simulink model "sys"  (R2024b) (continued)
== system sys == (continued)
  Subsystem5 : Subsystem  Commented=on
  Subsystem6 : Subsystem  Commented=on
      settings: PortSchema="{\"entries\":[{\"content\":{\"sides\":[{\"content\":{\"connectorIds\":[\"In1\",\"In2\"]},\"type\":\"ConnectorPlacement.EquallySpacedRectSide\",\"uuid\":\"1da95429-f45f-47f6-8988-21d04e9d7301\"},{\"content\":{\"connectorIds\":[\"In3\",\"In4\",\"Out1\",\"Out2\"],\"side\":\"RIGHT\"},\"type\":\"ConnectorPlacement.EquallySpacedRectSide\",\"uuid\":\"91f268de-d4f8-47bf-a89c-ce608da26a7f\"},{\"content\":{\"side\":\"TOP\"},\"type\":\"ConnectorPlacement.EquallySpacedRectSide\",\"uuid\":\"00e3ca0a-a055-4c98-9a5b-ca3cdd15cacc\"},{\"content\":{\"side\":\"BOTTOM\"},\"type\":\"ConnectorPlacement.EquallySpacedRectSide\",\"uuid\":\"425101d0-2a50-46ee-9f18-05564d8961df\"}]},\"type\":\"ConnectorPlacement.EquallySpacedConnector\",\"uuid\":\"7fddf75c-e649-487d-9520-ce1553949b22\"}],\"packageUris\":[\"http://schema.mathworks.com/mf0/Connector/R2020b\"],\"version\":\"1.0\"}"
  Subsystem7 : Subsystem  Commented=on
      settings: PortSchema="{\"entries\":[{\"content\":{\"sides\":[{\"content\":{\"connectorIds\":[\"In1\"]},\"type\":\"ConnectorPlacement.EquallySpacedRectSide\",\"uuid\":\"1da95429-f45f-47f6-8988-21d04e9d7301\"},{\"content\":{\"connectorIds\":[\"Out1\",\"In2\",\"In3\"],\"side\":\"RIGHT\"},\"type\":\"ConnectorPlacement.EquallySpacedRectSide\",\"uuid\":\"91f268de-d4f8-47bf-a89c-ce608da26a7f\"},{\"content\":{\"side\":\"TOP\"},\"type\":\"ConnectorPlacement.EquallySpacedRectSide\",\"uuid\":\"00e3ca0a-a055-4c98-9a5b-ca3cdd15cacc\"},{\"content\":{\"side\":\"BOTTOM\"},\"type\":\"ConnectorPlacement.EquallySpacedRectSide\",\"uuid\":\"425101d0-2a50-46ee-9f18-05564d8961df\"}]},\"type\":\"ConnectorPlacement.EquallySpacedConnector\",\"uuid\":\"7fddf75c-e649-487d-9520-ce1553949b22\"}],\"packageUris\":[\"http://schema.mathworks.com/mf0/Connector/R2020b\"],\"version\":\"1.0\"}"
  Subsystem8 : Subsystem  Commented=on
      settings: PortSchema="{\"entries\":[{\"content\":{\"sides\":[{\"content\":{\"connectorIds\":[\"In1\"]},\"type\":\"ConnectorPlacement.EquallySpacedRectSide\",\"uuid\":\"1da95429-f45f-47f6-8988-21d04e9d7301\"},{\"content\":{\"connectorIds\":[\"Out1\",\"In2\",\"In3\"],\"side\":\"RIGHT\"},\"type\":\"ConnectorPlacement.EquallySpacedRectSide\",\"uuid\":\"91f268de-d4f8-47bf-a89c-ce608da26a7f\"},{\"content\":{\"side\":\"TOP\"},\"type\":\"ConnectorPlacement.EquallySpacedRectSide\",\"uuid\":\"00e3ca0a-a055-4c98-9a5b-ca3cdd15cacc\"},{\"content\":{\"side\":\"BOTTOM\"},\"type\":\"ConnectorPlacement.EquallySpacedRectSide\",\"uuid\":\"425101d0-2a50-46ee-9f18-05564d8961df\"}]},\"type\":\"ConnectorPlacement.EquallySpacedConnector\",\"uuid\":\"7fddf75c-e649-487d-9520-ce1553949b22\"}],\"packageUris\":[\"http://schema.mathworks.com/mf0/Connector/R2020b\"],\"version\":\"1.0\"}"
  Subsystem9 : Subsystem  Commented=on
  Sum{,1,6,30} (4 alike) : Sum  Commented=on  Inputs=|+-
  Sum{10,13,18,21,33} (5 alike) : Sum  Commented=on  Inputs=+|+
  Sum{7..8,11..12,15,19,28..29,31,34..35} (11 alike) : Sum  Commented=on  Inputs=|++
  Sum{9,14,20,32} (4 alike) : Sum  Commented=on  Inputs=++|
  Sum16 : Sum  Commented=on  Inputs=|-+
  Sum17 : Sum  Commented=on  Inputs=|-+
  Sum2 : Sum  Inputs=|+-
  Sum3 : Sum  Inputs=++|
  Sum4 : Sum  Inputs=|++
  Sum5 : Sum  Inputs=|++
  Unit Delay : UnitDelay  Commented=on  SampleTime=0.01  HasFrameUpgradeWarning=on
  Unit Delay1 : UnitDelay  Commented=on  SampleTime=0.01  HasFrameUpgradeWarning=on
  Unit Delay{2..9} (8 alike) : UnitDelay  SampleTime=0.01  HasFrameUpgradeWarning=on
  Zero-Order Hold{1..8,11..14,17..30} (26 alike) : ZeroOrderHold  Commented=on  SampleTime=0.005
  Zero-Order Hold10 : ZeroOrderHold  SampleTime=0.005
  Zero-Order Hold9 : ZeroOrderHold  SampleTime=0.005
  roll环 : Subsystem  Commented=on
  roll环1 : Subsystem  Commented=on
  roll环2 : Subsystem  Commented=on
  roll环3 : Subsystem  Commented=on
      settings: PortSchema="{\"entries\":[{\"content\":{\"sides\":[{\"content\":{\"connectorIds\":[\"In1\"]},\"type\":\"ConnectorPlacement.EquallySpacedRectSide\",\"uuid\":\"c591d2ad-27e5-4316-87d8-5b4afe45ce5e\"},{\"content\":{\"connectorIds\":[\"Out1\",\"Out2\",\"In2\"],\"side\":\"RIGHT\"},\"type\":\"ConnectorPlacement.EquallySpacedRectSide\",\"uuid\":\"2c64d98f-78fa-4f7e-8b4c-47a380c9414e\"},{\"content\":{\"side\":\"TOP\"},\"type\":\"ConnectorPlacement.EquallySpacedRectSide\",\"uuid\":\"64da0bad-248b-47b6-8bff-ca1e26f13c40\"}]},\"type\":\"ConnectorPlacement.EquallySpacedConnector\",\"uuid\":\"aa7f0bc1-431c-4b94-aa14-1ec578d03f3c\"}],\"packageUris\":[\"http://schema.mathworks.com/mf0/Connector/R2020b\"],\"version\":\"1.0\"}"
  roll环4 : Subsystem
  x_d1 : Step  Commented=on  After=-1  SampleTime=0
  xd1 : Step  Commented=on  After=-1  SampleTime=0
  yaw环 : Subsystem  Commented=on
  yaw环1 : Subsystem  Commented=on
  yaw环2 : Subsystem  Commented=on
  yaw环3 : Subsystem  Commented=on
  yaw环4 : Subsystem
  双腿角度差环 : Subsystem  Commented=on
  双腿角度差环1 : Subsystem  Commented=on
  双腿角度差环2 : Subsystem  Commented=on
  双腿角度差环3 : Subsystem  Commented=on
  双腿角度差环4 : Subsystem
  wires:
  Demux5/1 -> Subsystem1/3(T3)
  Demux11/2 -> Subsystem9/4(T4)
  Subsystem9/8(L2) -> MATLAB Function15/3(l), Fl2/2(L1), MATLAB Function12/1(L)
  Saturation3/1 -> Demux7/1
  Demux10/1 -> Subsystem9/1(T1)
  Subsystem1/6(q3) -> MATLAB Function5/4(q1)
  Subsystem2/2(q1) -> MATLAB Function6/4(q1)
  Saturation9/1 -> Demux5/1
  Derivative6/1 -> Mux4/2
  Zero-Order Hold2/1 -> Saturation9/1
  Mux3/1 -> MATLAB Function9/1(State)
  Saturation6/1 -> Demux10/1
  Subsystem9/9(theta2) -> Derivative9/1, Mux6/1
  Demux8/2 -> Sum12/2
  Demux13/1 -> Zero-Order Hold18/1
  MATLAB Function6/1(T12) -> Zero-Order Hold3/1
  Fl1/1(F) -> MATLAB Function14/1(Fl)
  MATLAB Function7/1(T12) -> Zero-Order Hold4/1
  Fl2/1(F) -> MATLAB Function15/1(Fl)
  Mux6/1 -> MATLAB Function13/1(State)
  Subsystem2/9(L2) -> MATLAB Function10/1(L0), MATLAB Function7/3(l), Subsystem7/2(L1)
  MATLAB Function8/1(K) -> MATLAB Function9/2(K)
  Zero-Order Hold1/1 -> Saturation/1
  Saturation/1 -> Demux/1
  Subsystem4/2(F) -> MATLAB Function5/1(F)
  MATLAB Function17/1(T) -> Demux12/1
  Demux6/1 -> Subsystem2/1(T1)
  Demux9/1 -> Subsystem2/6(Tw2)
  Subsystem6/1(F) -> MATLAB Function/1(F)
  MATLAB Function12/1(K) -> MATLAB Function13/2(K)
  Subsystem9/4(theta1) -> Mux5/1, Derivative7/1
  MATLAB Function11/1(T) -> Demux9/1
  Subsystem9/10(q3) -> MATLAB Function15/4(q1)
  Subsystem6/2(T) -> MATLAB Function/2(T)
  Demux/2 -> Subsystem1/2(T2)
  Constant10/1 -> Subsystem4/2(L_d), Subsystem6/2(L_d)
  Derivative7/1 -> Mux5/2
  Zero-Order Hold6/1 -> Saturation5/1
  Constant11/1 -> Subsystem4/1(theta_d), Subsystem6/1(theta_d)
  Subsystem1/5(q2) -> MATLAB Function/5(q2)
  Subsystem1/4(q1) -> MATLAB Function/4(q1)
  Derivative9/1 -> Mux6/2
  Demux/1 -> Subsystem1/1(T1)
  MATLAB Function15/1(T12) -> Zero-Order Hold6/1
  Subsystem2/12(q4) -> MATLAB Function7/5(q2)
  Zero-Order Hold4/1 -> Saturation3/1
  Subsystem1/2(L1) -> Subsystem6/3(L1), MATLAB Function/3(l)
  Mux5/1 -> MATLAB Function17/1(State)
  Subsystem9/7(dphi) -> Mux5/6, Mux6/6
  Mux4/1 -> MATLAB Function11/1(State)
  Subsystem1/3(L2) -> MATLAB Function5/3(l), Subsystem4/3(L1)
  Subsystem2/1(q2) -> MATLAB Function6/5(q2)
  MATLAB Function13/1(T) -> Demux13/1
  Subsystem1/9(theta2) -> Subsystem4/4(theta1)
  Zero-Order Hold3/1 -> Saturation4/1
  MATLAB Function16/1(K) -> MATLAB Function17/2(K)
  Demux6/2 -> Subsystem2/2(T2)
  MATLAB Function14/1(T12) -> Zero-Order Hold5/1
  Subsystem4/1(T) -> MATLAB Function5/2(T)
  Subsystem2/3(L1) -> MATLAB Function6/3(l), Subsystem8/2(L1), MATLAB Function8/1(L0)
  Demux13/2 -> MATLAB Function15/2(Tp)
  Subsystem1/7(q4) -> MATLAB Function5/5(q2)
  Subsystem9/2(q1) -> MATLAB Function14/4(q1)
  Subsystem2/5(RobotAngle) -> Mux3/5, Mux4/5
  Demux10/2 -> Subsystem9/2(T2)
  Derivative/1 -> Mux3/2
  Saturation5/1 -> Demux11/1
  Zero-Order Hold5/1 -> Saturation6/1
  Subsystem2/11(q3) -> MATLAB Function7/4(q1)
  Subsystem9/11(q4) -> MATLAB Function15/5(q2)
  Subsystem2/4(theta1) -> Subsystem8/3(theta), Derivative/1, Mux3/1
  MATLAB Function/1(T12) -> Zero-Order Hold1/1
  Demux12/1 -> Zero-Order Hold17/1
  Demux5/2 -> Subsystem1/4(T4)
  MATLAB Function10/1(K) -> MATLAB Function11/2(K)
  Demux12/2 -> MATLAB Function14/2(Tp)
  Demux7/2 -> Subsystem2/4(T4)
  Demux9/2 -> Sum13/2
  Subsystem9/1(q2) -> MATLAB Function14/5(q2)
  Demux8/1 -> Subsystem2/5(Tw1), Scope/1
  Demux7/1 -> Subsystem2/3(T3)
  Subsystem1/8(theta1) -> Subsystem6/4(theta1)
  Constant2/1 -> Fl2/1(L_d), Fl1/1(L_d)
  Subsystem2/6(RobotAngleSpeed) -> Mux3/6, Mux4/6
  MATLAB Function9/1(T) -> Demux8/1
  Saturation4/1 -> Demux6/1
  Demux11/1 -> Subsystem9/3(T3)
  MATLAB Function5/1(T12) -> Zero-Order Hold2/1
  Subsystem9/3(L1) -> MATLAB Function14/3(l), Fl1/2(L1), MATLAB Function16/1(L)
  Derivative1/1 -> Mux5/4
  Derivative2/1 -> Mux6/4
  Add/1 -> Mux5/3, Derivative1/1
  Subsystem9/5(x1) -> Add/2
  Step9/1 -> Integrator1/1
  Integrator1/1 -> Add/1
  Step8/1 -> Integrator2/1
  Sum/1 -> Derivative2/1, Mux6/3
  Subsystem9/12(x2) -> Sum/1
  Integrator2/1 -> Sum/2
  Sine Wave16/1 -> Subsystem7/1(L_d), Subsystem8/1(L_d)
  Sum1/1 -> Derivative10/1, Mux2/3
  Add1/1 -> Mux1/3, Derivative3/1
  Demux3/1 -> Subsystem3/6(Tw2)
  Subsystem3/8(phi) -> Mux1/5, Mux2/5
  Mux2/1 -> MATLAB Function2/1(State)
  Derivative12/1 -> Mux2/2
  Derivative3/1 -> Mux1/4
  Constant8/1 -> Integrator4/1
  MATLAB Function4/1(T) -> Demux1/1
  Subsystem3/9(dphi) -> Mux1/6, Mux2/6
  MATLAB Function1/1(K) -> MATLAB Function2/2(K)
  MATLAB Function2/1(T) -> Demux3/1
  Integrator3/1 -> Add1/1
  Subsystem3/4(theta1) -> Mux1/1, Derivative11/1
  MATLAB Function3/1(K) -> MATLAB Function4/2(K)
  Constant7/1 -> Integrator3/1
  Derivative10/1 -> Mux2/4
  Subsystem3/6(L2) -> MATLAB Function19/3(l), Fl4/2(L1), MATLAB Function1/1(L)
  Subsystem3/5(x1) -> Add1/2
  Subsystem3/12(x2) -> Sum1/1
  Mux1/1 -> MATLAB Function4/1(State)
  Subsystem3/7(theta2) -> Derivative12/1, Mux2/1
  Demux1/1 -> Subsystem3/5(Tw1)
  Integrator4/1 -> Sum1/2
  Derivative11/1 -> Mux1/2
  Constant9/1 -> Fl4/1(L_d), Fl3/1(L_d)
  MATLAB Function18/1(T12) -> Zero-Order Hold7/1
  Demux14/2 -> Subsystem3/4(T4)
  Demux4/2 -> Subsystem3/2(T2)
  Demux4/1 -> Subsystem3/1(T1)
  Demux14/1 -> Subsystem3/3(T3)
  Zero-Order Hold8/1 -> Saturation1/1
  MATLAB Function19/1(T12) -> Zero-Order Hold8/1
  Subsystem3/10(q3) -> MATLAB Function19/4(q1)
  Subsystem3/1(q2) -> MATLAB Function18/5(q2)
  Fl4/1(F) -> MATLAB Function19/1(Fl)
  Fl3/1(F) -> MATLAB Function18/1(Fl)
  Saturation1/1 -> Demux14/1
  Zero-Order Hold7/1 -> Saturation2/1
  Subsystem3/2(q1) -> MATLAB Function18/4(q1)
  Subsystem3/11(q4) -> MATLAB Function19/5(q2)
  Demux1/2 -> MATLAB Function18/2(Tp)
  Demux3/2 -> MATLAB Function19/2(Tp)
  Saturation2/1 -> Demux4/1
  Subsystem3/3(L1) -> Fl3/2(L1), MATLAB Function18/3(l), MATLAB Function3/1(L)
  Subsystem10/3(L1) -> MATLAB Function24/1(L), MATLAB Function21/3(l), Fl5/2(L1)
  Subsystem10/5(x1) -> Add2/2
  MATLAB Function23/1(T) -> Demux17/1
  Add2/1 -> Mux7/3, Derivative16/1
  Subsystem10/2(q1) -> MATLAB Function21/4(q1)
  MATLAB Function20/1(K) -> MATLAB Function23/2(K)
  Subsystem10/6(L2) -> Fl6/2(L1), MATLAB Function22/3(l), MATLAB Function20/1(L)
  Subsystem10/10(q3) -> MATLAB Function22/4(q1)
  MATLAB Function24/1(K) -> MATLAB Function25/2(K)
  MATLAB Function21/1(T12) -> Zero-Order Hold9/1
  Demux17/1 -> Subsystem10/6(Tw2)
  Sum2/1 -> Derivative13/1, Mux8/3
  Mux8/1 -> MATLAB Function23/1(State)
  Saturation8/1 -> Demux18/1
  Zero-Order Hold10/1 -> Saturation7/1
  Derivative15/1 -> Mux8/2
  Subsystem10/1(q2) -> MATLAB Function21/5(q2)
  Subsystem10/12(x2) -> Sum2/1
  MATLAB Function22/1(T12) -> Zero-Order Hold10/1
  Integrator5/1 -> Add2/1
  Derivative16/1 -> Mux7/4
  Derivative13/1 -> Mux8/4
  Demux15/2 -> MATLAB Function21/2(Tp)
  Mux7/1 -> MATLAB Function25/1(State)
  Integrator6/1 -> Sum2/2
  Demux16/2 -> Subsystem10/4(T4)
  MATLAB Function25/1(T) -> Demux15/1
  Constant5/1 -> Integrator5/1
  Demux16/1 -> Subsystem10/3(T3)
  Demux15/1 -> Subsystem10/5(Tw1)
  Fl6/1(F) -> MATLAB Function22/1(Fl)
  Derivative14/1 -> Mux7/2
  Zero-Order Hold9/1 -> Saturation8/1
  Demux18/2 -> Subsystem10/2(T2)
  Fl5/1(F) -> MATLAB Function21/1(Fl)
  Constant6/1 -> Integrator6/1
  Subsystem10/9(dphi) -> Mux8/6, Mux7/6
  Constant13/1 -> Fl6/1(L_d), Fl5/1(L_d)
  Subsystem10/11(q4) -> MATLAB Function22/5(q2)
  Demux17/2 -> MATLAB Function22/2(Tp)
  Demux18/1 -> Subsystem10/1(T1)
  Saturation7/1 -> Demux16/1
  Subsystem10/8(phi) -> Sum3/2
  Constant12/1 -> Sum3/1
  Sum3/1 -> Mux7/5, Mux8/5
  Subsystem10/4(theta1) -> Sum4/1
  Sum4/1 -> Mux7/1, Derivative14/1
  Constant14/1 -> Sum4/2
  Subsystem10/7(theta2) -> Sum5/1
  Constant15/1 -> Sum5/2
  Sum5/1 -> Mux8/1, Derivative15/1
  Subsystem2/7(x1) -> Derivative4/1, Mux3/3
  Derivative4/1 -> Mux3/4
  Subsystem2/8(x2) -> Derivative5/1, Mux4/3
  Derivative5/1 -> Mux4/4
  Subsystem2/10(theta2) -> Derivative6/1, Mux4/1
  Sum12/1 -> MATLAB Function6/2(Tp)
  Sum13/1 -> MATLAB Function7/2(Tp)
  Fl10/1(F) -> MATLAB Function34/1(Fl)
  Demux23/1 -> EKF_x5/4(T1), Sum28/2
  Subsystem14/4(theta1) -> 双腿角度差环/1(theta1), Sum16/1
  Constant26/1 -> Sum17/2
  Subsystem14/7(L2) -> MATLAB Function32/1(L), MATLAB Function34/3(l), Fl10/3(L1)
  MATLAB Function36/1(K) -> MATLAB Function37/2(K)
  Demux26/2 -> Subsystem14/2(T2)
  Sum17/1 -> Mux12/1, Derivative22/1
  Subsystem14/5(dphi) -> Mux12/6, Mux11/6
  Zero-Order Hold14/1 -> Demux26/1
  Subsystem14/8(theta2) -> Sum17/1, 双腿角度差环/2(theta2)
  MATLAB Function32/1(K) -> MATLAB Function35/2(K)
  Mux11/1 -> Scope5/1, MATLAB Function37/1(State)
  Subsystem14/3(L1) -> MATLAB Function33/3(l), Fl9/3(L1), EKF_x5/3(L), MATLAB Function36/1(L)
  Subsystem14/1(q2) -> MATLAB Function33/5(q2)
  Subsystem14/10(q4) -> MATLAB Function34/5(q2)
  Demux24/1 -> Subsystem14/3(T3)
  MATLAB Function37/1(T) -> Demux23/1
  Constant25/1 -> Sum16/2
  Demux25/1 -> EKF_x5/6(T2), Sum29/2
  Subsystem14/11(droll) -> Fl9/4(droll), Fl10/4(droll), roll环/1(droll)
  MATLAB Function34/1(T12) -> Zero-Order Hold13/1
  Derivative22/1 -> Mux12/2
  Demux24/2 -> Subsystem14/4(T4)
  Subsystem14/2(q1) -> MATLAB Function33/4(q1)
  Subsystem14/9(q3) -> MATLAB Function34/4(q1)
  MATLAB Function33/1(T12) -> Zero-Order Hold14/1
  Fl9/1(F) -> MATLAB Function33/1(Fl)
  Demux26/1 -> Scope23/1, Subsystem14/1(T1)
  Subsystem14/6(phi) -> Mux11/5, Mux12/5
  Sum16/1 -> Mux11/1, Derivative21/1
  Mux12/1 -> MATLAB Function35/1(State)
  Zero-Order Hold13/1 -> Demux24/1
  Derivative21/1 -> Mux11/2
  MATLAB Function35/1(T) -> Demux25/1
  Subsystem14/12(dyaw) -> yaw环/1(dyaw)
  Sum21/1 -> MATLAB Function34/2(Tp)
  Sum20/1 -> MATLAB Function33/2(Tp)
  Sum30/1 -> Mux5/5, Mux6/5
  Subsystem9/6(phi) -> Sum30/1
  Step7/1 -> Sum30/2
  Saturation14/1 -> Scope4/1, Subsystem9/5(Tw1)
  Zero-Order Hold17/1 -> Saturation14/1
  Zero-Order Hold18/1 -> Saturation15/1
  Saturation15/1 -> Scope3/1, Subsystem9/6(Tw2)
  Sum28/1 -> Zero-Order Hold19/1
  Zero-Order Hold19/1 -> Subsystem14/5(Tw1), Scope24/1
  Zero-Order Hold20/1 -> Subsystem14/6(Tw2)
  Sum29/1 -> Zero-Order Hold20/1
  Step3/1 -> Rate Limiter3/1
  Constant22/1 -> Fl9/1(L_d), Fl10/1(L_d)
  roll环1/1(Tr1) -> Fl8/2(Tr1)
  Step4/1 -> Rate Limiter4/1
  Constant17/1 -> Fl8/1(L_d), Fl7/1(L_d)
  Step11/1 -> Rate Limiter5/1
  双腿角度差环1/2(dT2) -> Sum10/1
  yaw环1/2(Ty2) -> Sum15/1
  Subsystem11/12(x2) -> Sum6/1
  Sum15/1 -> Zero-Order Hold22/1
  Subsystem11/7(phi) -> Mux10/5, Mux9/5
  Saturation20/1 -> Subsystem11/5(Tw1)
  Subsystem11/5(x1) -> Add3/2
  Saturation21/1 -> Subsystem11/6(Tw2)
  双腿角度差环1/1(dT1) -> Sum9/1
  x_d1/1 -> Integrator12/1
  Zero-Order Hold22/1 -> Saturation21/1
  Demux20/1 -> Subsystem11/3(T3)
  Subsystem11/4(theta1) -> 双腿角度差环1/1(theta1), Sum7/1
  Subsystem11/14(dyaw) -> yaw环1/1(dyaw)
  Sum8/1 -> Mux10/1, Derivative18/1
  Subsystem11/9(theta2) -> 双腿角度差环1/2(theta2), Sum8/1
  yaw环1/1(Ty1) -> Sum11/1
  Fl7/1(F) -> MATLAB Function28/1(Fl)
  Mux9/1 -> MATLAB Function31/1(State)
  xd1/1 -> Integrator11/1
  Demux22/2 -> Subsystem11/2(T2)
  Derivative19/1 -> Mux9/4
  Saturation10/1 -> Demux20/1
  Subsystem11/13(droll) -> Fl8/4(droll), Fl7/4(droll), roll环1/1(droll)
  Sum7/1 -> Mux9/1, Derivative17/1
  Subsystem11/8(L2) -> MATLAB Function28/3(l), Fl7/3(L1), MATLAB Function26/1(L)
  Zero-Order Hold11/1 -> Saturation10/1
  roll环1/2(Tr2) -> Fl7/2(Tr2)
  Add3/1 -> Mux9/3, Derivative19/1
  Mux10/1 -> MATLAB Function29/1(State)
  Demux21/1 -> Sum15/2
  Zero-Order Hold12/1 -> Saturation11/1
  Sum6/1 -> Derivative20/1, Mux10/3
  Subsystem11/6(dphi) -> Mux10/6, Mux9/6
  Demux19/2 -> Sum9/2
  MATLAB Function29/1(T) -> Demux21/1
  Subsystem11/10(q3) -> MATLAB Function28/4(q1)
  MATLAB Function28/1(T12) -> Zero-Order Hold11/1
  MATLAB Function30/1(K) -> MATLAB Function31/2(K)
  Sum10/1 -> MATLAB Function28/2(Tp)
  MATLAB Function27/1(T12) -> Zero-Order Hold12/1
  Saturation11/1 -> Demux22/1
  Demux22/1 -> Subsystem11/1(T1)
  Subsystem11/11(q4) -> MATLAB Function28/5(q2)
  Subsystem11/2(q1) -> MATLAB Function27/4(q1)
  Constant18/1 -> Sum7/2
  Subsystem11/1(q2) -> MATLAB Function27/5(q2)
  Sum9/1 -> MATLAB Function27/2(Tp)
  Derivative20/1 -> Mux10/4
  MATLAB Function26/1(K) -> MATLAB Function29/2(K)
  Derivative17/1 -> Mux9/2
  Demux21/2 -> Sum10/2
  MATLAB Function31/1(T) -> Demux19/1
  Demux19/1 -> Sum11/2
  Zero-Order Hold21/1 -> Saturation20/1
  Derivative18/1 -> Mux10/2
  Subsystem11/3(L1) -> MATLAB Function27/3(l), Fl8/3(L1), MATLAB Function30/1(L)
  Fl8/1(F) -> MATLAB Function27/1(Fl)
  Sum11/1 -> Zero-Order Hold21/1
  Demux20/2 -> Subsystem11/4(T4)
  Rate Limiter7/1 -> yaw环1/2(yaw_d)
  Constant19/1 -> Sum8/2
  Constant20/1 -> roll环1/2(roll_d)
  Subsystem12/2(q1) -> MATLAB Function45/4(q1)
  Fl13/1(F) -> MATLAB Function46/1(Fl), MATLAB Function47/2(F)
  MATLAB Function45/1(T12) -> Zero-Order Hold24/1
  Subsystem12/9(q3) -> MATLAB Function46/4(q1)
  Sum33/1 -> MATLAB Function47/3(Tp), MATLAB Function46/2(Tp)
  Zero-Order Hold26/1 -> Subsystem12/6(Tw2)
  Subsystem12/3(L1) -> EKF_x1/3(L), Mux/3, MATLAB Function45/3(l), Fl14/3(L1)
  Demux32/1 -> Subsystem12/3(T3)
  Derivative29/1 -> Mux16/2
  Demux32/2 -> Subsystem12/4(T4)
  Subsystem12/1(q2) -> MATLAB Function45/5(q2)
  Step14/1 -> Rate Limiter9/1
  Subsystem12/8(theta2) -> Derivative31/1, Derivative30/1, MATLAB Function47/7(theta), 双腿角度差环2/2(theta2), Derivative29/1, Mux16/1
  MATLAB Function46/1(T12) -> Zero-Order Hold23/1
  Saturation22/1 -> Demux32/1
  Subsystem12/4(theta1) -> Mux15/1, Derivative28/1, 双腿角度差环2/1(theta1)
  Subsystem12/6(phi) -> Mux16/5, Mux15/5
  Zero-Order Hold25/1 -> Subsystem12/5(Tw1)
  Subsystem12/10(q4) -> MATLAB Function46/5(q2)
  Subsystem12/5(dphi) -> Mux16/6, Mux15/6
  Sum34/1 -> Zero-Order Hold25/1
  Subsystem12/7(L2) -> Mux18/3, Derivative23/1, Derivative8/1, MATLAB Function47/4(L0), MATLAB Function46/3(l), Fl13/3(L1)
  Demux34/1 -> Subsystem12/1(T1)
  Derivative28/1 -> Mux15/2
  Demux34/2 -> Subsystem12/2(T2)
  Subsystem12/12(dyaw) -> yaw环2/1(dyaw)
  Demux37/1 -> Sum34/2, EKF_x1/4(T1)
  Subsystem12/11(droll) -> Fl14/4(droll), Fl13/4(droll), roll环2/1(droll)
  Saturation23/1 -> Demux34/1
  Constant27/1 -> Fl14/1(L_d), Fl13/1(L_d)
  Sum35/1 -> Zero-Order Hold26/1
  Sum32/1 -> MATLAB Function45/2(Tp)
  Constant29/1 -> roll环2/2(roll_d)
  Zero-Order Hold23/1 -> Saturation22/1
  Zero-Order Hold24/1 -> Saturation23/1
  Step2/1 -> Rate Limiter8/1
  Mux15/1 -> Mux/1, Demux35/1
  Mux/1 -> Rate Transition1/1
  Mux17/1 -> Mux18/2, Mux/2
  Mux16/1 -> Mux18/1
  Rate Transition1/1 -> Interpreted MATLAB Function/1
  Rate Transition2/1 -> Interpreted MATLAB Function1/1
  roll环2/1(Tr1) -> Fl14/2(Tr1)
  roll环2/2(Tr2) -> Fl13/2(Tr2)
  双腿角度差环2/2(dT2) -> Sum32/1
  双腿角度差环2/1(dT1) -> Sum33/1
  Demux23/2 -> Gain1/1
  Rate Limiter2/1 -> roll环/2(roll_d)
  Constant3/1 -> Rate Limiter2/1
  Gain1/1 -> EKF_x5/5(Tp1), Sum20/2
  roll环/1(Tr1) -> Fl9/2(Tr1)
  双腿角度差环/1(dT1) -> Sum20/1
  双腿角度差环/2(dT2) -> Sum21/1
  roll环/2(Tr2) -> Fl10/2(Tr2)
  Demux25/2 -> EKF_x5/7(Tp2), Gain2/1
  Gain2/1 -> Sum21/2
  Subsystem12/15(dx_w) -> EKF_x1/2(dx_w)
  Subsystem12/14(acc_x) -> EKF_x1/1(acc_x)
  Demux37/2 -> EKF_x1/6(Tp1), Sum32/2
  EKF_x1/1(x_hat) -> Mux16/3, Mux15/3
  Mux19/1 -> Scope9/1
  Demux36/1 -> Mux19/2
  Mux20/1 -> Scope9/2
  Mux21/1 -> Scope9/3
  Mux22/1 -> Scope9/4
  Mux23/1 -> Scope9/5
  Mux24/1 -> Scope9/6
  Demux35/1 -> Mux19/1
  Demux35/2 -> Mux20/1
  Demux35/3 -> Mux21/1
  Demux35/4 -> Mux22/1
  Demux35/5 -> Mux23/1
  Demux35/6 -> Mux24/1
  Demux36/2 -> Mux20/2
  Demux36/3 -> Mux21/2
  Demux36/4 -> Mux22/2
  Demux36/5 -> Mux23/2
  Demux36/6 -> Mux24/2
  Mux18/1 -> Rate Transition2/1
  Constant/1 -> Mux17/3, Mux17/6, Mux17/5, Mux17/2, Mux17/1
  EKF_x1/2(dx_hat) -> Mux16/4, Mux15/4, Scope2/2, Scope6/1
  Step/1 -> Mux17/4
  Subsystem12/13(ay) -> MATLAB Function47/1(ddz_M)
  Derivative8/1 -> MATLAB Function47/5(L0_dot)
  Derivative23/1 -> MATLAB Function47/6(L0_ddot)
  Derivative30/1 -> MATLAB Function47/8(dtheta)
  Derivative31/1 -> MATLAB Function47/9(ddtheta)
  Step15/1 -> Rate Limiter10/1
  Constant23/1 -> yaw环2/2(yaw_d)
  Unit Delay/1 -> Scope14/1, Subsystem12/7(FN), Mux18/4, Mux/4
  Fl14/1(F) -> MATLAB Function45/1(Fl)
  EKF_x1/3(Out1) -> Integrator/1
  Integrator/1 -> Scope2/3
  EKF_x1/4(Out2) -> Scope2/4
  Integrator9/1 -> Scope2/1
  Subsystem12/16(Out1) -> Integrator9/1
  MATLAB Function47/1(F_N) -> Unit Delay/1
  Subsystem8/1(F) -> MATLAB Function6/1(F)
  Subsystem7/1(F) -> MATLAB Function7/1(F)
  Interpreted MATLAB Function/1 -> Demux37/1
  Mux36/1 -> Demux36/1
  Demux37/3 -> Mux36/1
  Demux37/4 -> Mux36/2
  Demux37/5 -> Mux36/3
  Demux37/6 -> Mux36/4
  Demux37/7 -> Mux36/5
  Demux37/8 -> Mux36/6
  Interpreted MATLAB Function1/1 -> Demux31/1
  Demux31/1 -> Sum35/2, EKF_x1/5(T2)
  Demux31/2 -> Sum33/2, EKF_x1/7(Tp2)
  Demux42/4 -> Mux37/2
  Demux42/8 -> Mux37/6
  Demux33/2 -> Sum18/2, EKF_x2/7(Tp2)
  Interpreted MATLAB Function3/1 -> Demux33/1
  Demux42/2 -> EKF_x2/6(Tp1), Sum14/2
  Subsystem13/9(q3) -> MATLAB Function48/4(q1)
  Fl16/1(F) -> MATLAB Function44/1(Fl)
  Subsystem13/10(q4) -> MATLAB Function48/5(q2)
  Zero-Order Hold30/1 -> Subsystem13/6(Tw2)
  Demux39/2 -> Subsystem13/2(T2)
  EKF_x2/2(dx_hat) -> Mux27/4, Mux26/4, Scope8/2, Scope10/1
  Demux41/2 -> Mux31/2
  Demux40/6 -> Mux35/1
  Sum19/1 -> Zero-Order Hold29/1
  Derivative33/1 -> Mux26/2
  Demux41/4 -> Mux33/2
  Subsystem13/12(dyaw) -> yaw环3/1(dyaw)
  EKF_x2/4(Out2) -> Scope8/4
  Demux38/2 -> Subsystem13/4(T4)
  Derivative32/1 -> MATLAB Function49/6(L0_ddot)
  Subsystem13/3(L1) -> EKF_x2/3(L), Mux25/3, MATLAB Function44/3(l), Fl16/3(L1)
  Demux33/1 -> Sum31/2, EKF_x2/5(T2)
  Demux42/5 -> Mux37/3
  Zero-Order Hold28/1 -> Demux39/1
  Demux42/6 -> Mux37/4
  Demux42/1 -> Sum19/2, EKF_x2/4(T1)
  MATLAB Function48/1(T12) -> Zero-Order Hold27/1
  Demux39/1 -> Subsystem13/1(T1)
  EKF_x2/1(x_hat) -> Mux27/3, Mux26/3
  Subsystem13/13(ay) -> MATLAB Function49/1(ddz_M)
  Subsystem13/15(dx_w) -> EKF_x2/2(dx_w)
  Mux25/1 -> Rate Transition3/1
  Demux40/3 -> Mux32/1
  Rate Transition4/1 -> Interpreted MATLAB Function3/1
  Subsystem13/11(droll) -> Fl16/4(droll), Fl15/4(droll), roll环3/1(droll)
  Mux35/1 -> Scope11/6
  Subsystem13/16(Out1) -> Integrator13/1
  Sum14/1 -> MATLAB Function44/2(Tp)
  Subsystem13/14(acc_x) -> EKF_x2/1(acc_x)
  Mux26/1 -> Mux25/1, Demux40/1
  Zero-Order Hold27/1 -> Demux38/1
  Mux31/1 -> Scope11/2
  Subsystem13/2(q1) -> MATLAB Function44/4(q1)
  Subsystem13/5(dphi) -> Mux27/6, Mux26/6
  Demux41/3 -> Mux32/2
  Demux38/1 -> Subsystem13/3(T3)
  Mux27/1 -> Mux29/1
  Demux40/4 -> Mux33/1
  Demux41/1 -> Mux30/2
  EKF_x2/3(Out1) -> Integrator10/1
  Subsystem13/7(L2) -> Mux29/3, Derivative32/1, Derivative37/1, MATLAB Function49/4(L0), MATLAB Function48/3(l), Fl15/3(L1)
  MATLAB Function49/1(F_N) -> Unit Delay1/1
  Mux33/1 -> Scope11/4
  Mux34/1 -> Scope11/5
  Demux42/7 -> Mux37/5
  Sum18/1 -> MATLAB Function49/3(Tp), MATLAB Function48/2(Tp)
  Interpreted MATLAB Function2/1 -> Demux42/1
  Mux30/1 -> Scope11/1
  Subsystem13/8(theta2) -> Derivative36/1, Derivative35/1, MATLAB Function49/7(theta), 双腿角度差环3/2(theta2), Derivative34/1, Mux27/1
  Subsystem13/4(theta1) -> Mux26/1, Derivative33/1, 双腿角度差环3/1(theta1)
  Mux32/1 -> Scope11/3
  Derivative37/1 -> MATLAB Function49/5(L0_dot)
  Mux37/1 -> Demux41/1
  Zero-Order Hold29/1 -> Subsystem13/5(Tw1)
  Demux40/2 -> Mux31/1
  MATLAB Function44/1(T12) -> Zero-Order Hold28/1
  Unit Delay1/1 -> Scope1/1, Subsystem13/7(FN), Mux29/4, Mux25/4
  Mux28/1 -> Mux29/2, Mux25/2
  Subsystem13/1(q2) -> MATLAB Function44/5(q2)
  Subsystem13/6(phi) -> Mux27/5, Mux26/5
  Demux40/5 -> Mux34/1
  Derivative36/1 -> MATLAB Function49/9(ddtheta)
  Integrator13/1 -> Scope8/1
  Demux40/1 -> Mux30/1
  Integrator10/1 -> Scope8/3
  Mux29/1 -> Rate Transition4/1
  Fl15/1(F) -> MATLAB Function49/2(F), MATLAB Function48/1(Fl)
  Derivative35/1 -> MATLAB Function49/8(dtheta)
  Derivative34/1 -> Mux27/2
  Demux41/5 -> Mux34/2
  Demux41/6 -> Mux35/2
  Rate Transition3/1 -> Interpreted MATLAB Function2/1
  Constant24/1 -> Mux28/1, Mux28/3, Mux28/6, Mux28/5, Mux28/2
  Sum31/1 -> Zero-Order Hold30/1
  Demux42/3 -> Mux37/1
  MATLAB Function50/1(y) -> Rate Limiter/1
  Clock/1 -> MATLAB Function50/2(t)
  yaw环3/3(forth) -> 双腿角度差环3/3(forth)
  Step16/1 -> Mux28/4
  Subsystem14/13(dx_w) -> EKF_x5/2(dx_w)
  Subsystem14/14(dx_a) -> EKF_x5/1(acc_x)
  EKF_x5/1(x_hat) -> Add4/1
  Step12/1 -> Rate Limiter22/1
  EKF_x5/2(dx_hat) -> Mux12/4, Mux11/4
  Add4/1 -> Mux12/3, Mux11/3
  Rate Limiter22/1 -> Add4/2
  yaw环/1(Ty1) -> Sum28/1
  yaw环/2(Ty2) -> Sum29/1
  Sine Wave/1 -> Rate Limiter6/1
  Constant16/1 -> yaw环/2(yaw_d)
  Rate Limiter11/1 -> MATLAB Function50/1(u)
  yaw环3/1(Ty1) -> Sum19/1
  yaw环3/2(Ty2) -> Sum31/1
  双腿角度差环3/1(dT1) -> Sum14/1
  双腿角度差环3/2(dT2) -> Sum18/1
  Step19/1 -> Rate Limiter12/1
  Constant34/1 -> Fl16/1(H_d), Fl15/1(H_d)
  roll环3/1(Tr1) -> Fl16/2(Tr1)
  roll环3/2(Tr2) -> Fl15/2(Tr2)
  Constant35/1 -> roll环3/2(roll_d)
  Rate Limiter/1 -> yaw环3/2(yaw_d)
  Step20/1 -> Rate Limiter11/1
  Mux43/1 -> Scope16/1
  Mux48/1 -> Scope16/6
  MATLAB Function54/1(y) -> Rate Limiter13/1
  Rate Transition5/1 -> Interpreted MATLAB Function4/1
  Mux44/1 -> Scope16/2
  Mux45/1 -> Scope16/3
  Mux46/1 -> Scope16/4
  Step18/1 -> MATLAB Function54/1(u)
  Clock1/1 -> MATLAB Function54/2(t)
  Mux47/1 -> Scope16/5
  Subsystem15/2(q1) -> [q1]
  Subsystem15/1(q2) -> [q2]
  Subsystem15/3(L1) -> [L1]
  Subsystem15/5(dphi) -> [dphi]
  Subsystem15/6(phi) -> [phi]
  Subsystem15/7(L2) -> [L2]
  Subsystem15/8(theta2) -> Derivative45/1, [theta2]
  Subsystem15/9(q3) -> [q3]
  Subsystem15/10(q4) -> [q4]
  [theta1] -> Mux38/1
  Derivative44/1 -> [dtheta1]
  Subsystem15/4(theta1) -> Derivative44/1, [theta1]
  Derivative45/1 -> [dtheta2]
  [dtheta1] -> Mux38/2
  EKF1/1(x_hat1) -> [x_hat1]
  EKF1/2(dx_hat1) -> [dx_hat1]
  Subsystem15/11(dx_w) -> [dx_w]
  Subsystem15/12(acc_x) -> [acc_x]
  Subsystem15/13(ay) -> [ay]
  Subsystem15/14(dyaw) -> [dyaw]
  Subsystem15/15(droll) -> [droll]
  [x_hat1] -> Mux38/3
  [dx_hat1] -> Mux38/4
  [phi] -> Mux38/5
  [dphi] -> Mux38/6
  [acc_x] -> EKF1/1(acc_x)
  [dx_w] -> Unit Delay6/1
  [L1] -> EKF1/3(L1)
  Mux38/1 -> Rate Transition5/1
  Demux46/1 -> [Tw1]
  Demux46/2 -> [Tp1]
  [Tw_1] -> Unit Delay4/1
  [Tp_1] -> Unit Delay5/1
  Subsystem/1(x_ref) -> Mux45/1, Mux41/3
  Subsystem/2(x_dot_ref) -> Mux46/1, Mux41/4
  Mux41/1 -> [X_ref]
  [X_ref] -> Mux38/7
  Interpreted MATLAB Function4/1 -> Demux46/1
  [L1] -> Mux38/8
  Delay/1 -> Mux38/9
  MATLAB Function53/1(F_N) -> Unit Delay2/1
  [F_N1] -> Delay/1
  [L2] -> Mux39/8
  [phi] -> Mux39/5
  [dphi] -> Mux39/6
  [F_N2] -> Delay1/1
  Demux43/1 -> [Tw2]
  Interpreted MATLAB Function5/1 -> Demux43/1
  [theta2] -> Mux39/1
  [X_ref] -> Mux39/7
  [x_hat2] -> Mux39/3
  Mux39/1 -> Rate Transition6/1
  Demux43/2 -> [Tp2]
  Delay1/1 -> Mux39/9
  [dx_hat2] -> Mux39/4
  [dtheta2] -> Mux39/2
  Rate Transition6/1 -> Interpreted MATLAB Function5/1
  [ay] -> MATLAB Function53/1(ddz_M)
  [dyaw] -> yaw环4/1(dyaw)
  [droll] -> Fl17/5(droll), Fl18/1(droll), roll环4/1(droll)
  roll环4/1(Hc1) -> Fl18/5(Hc1)
  roll环4/2(Hc2) -> Fl17/2(Hc2)
  [L2] -> Fl17/3(L2)
  [L1] -> Fl18/3(L1)
  Constant38/1 -> [H_d]
  [H_d] -> Fl18/4(H_d)
  [H_d] -> Fl17/1(H_d)
  Fl18/1(F1) -> [F1]
  Fl17/1(F2) -> [F2]
  [theta1] -> 双腿角度差环4/1(theta1)
  [theta2] -> 双腿角度差环4/2(theta2)
  yaw环4/1(Twy1) -> [Twy1]
  yaw环4/2(Twy2) -> [Twy2]
  yaw环4/3(forth) -> [forth]
  [forth] -> 双腿角度差环4/3(forth)
  双腿角度差环4/1(Tpy1) -> [Tpy1]
  双腿角度差环4/2(Tpy2) -> [Tpy2]
  [F1] -> MATLAB Function51/1(Fl)
  [L1] -> MATLAB Function51/3(l)
  [q1] -> MATLAB Function51/4(q1)
  [q2] -> MATLAB Function51/5(q2)
  MATLAB Function51/1(T1) -> [T1]
  MATLAB Function51/2(T2) -> [T2]
  MATLAB Function52/1(T1) -> [T3]
  [q3] -> MATLAB Function52/4(q1)
  [L2] -> MATLAB Function52/3(l)
  [F2] -> MATLAB Function52/1(Fl)
  MATLAB Function52/2(T2) -> [T4]
  [q4] -> MATLAB Function52/5(q2)
  [F1] -> MATLAB Function53/2(F)
  [Tpy2] -> Add6/2
  [Tp2] -> Add6/1
  Add6/1 -> [Tp_2]
  [Tp1] -> Add5/1
  [Tpy1] -> Add5/2
  Add5/1 -> [Tp_1]
  [Tp_1] -> MATLAB Function51/2(Tp)
  [Tp_2] -> MATLAB Function52/2(Tp)
  [Tp_1] -> MATLAB Function53/3(Tp)
  [L1] -> MATLAB Function53/4(L0), Derivative38/1
  Derivative38/1 -> MATLAB Function53/5(L0_dot), Derivative39/1
  Derivative39/1 -> MATLAB Function53/6(L0_ddot)
  [theta1] -> Derivative40/1, MATLAB Function53/7(theta)
  Derivative41/1 -> MATLAB Function53/9(ddtheta)
  Derivative40/1 -> MATLAB Function53/8(dtheta), Derivative41/1
  [F2] -> MATLAB Function55/2(F)
  [theta2] -> Derivative46/1, MATLAB Function55/7(theta)
  [ay] -> MATLAB Function55/1(ddz_M)
  Derivative46/1 -> MATLAB Function55/8(dtheta), Derivative47/1
  MATLAB Function55/1(F_N) -> Unit Delay3/1
  Derivative47/1 -> MATLAB Function55/9(ddtheta)
  [L2] -> MATLAB Function55/4(L0), Derivative42/1
  Derivative42/1 -> MATLAB Function55/5(L0_dot), Derivative43/1
  Derivative43/1 -> MATLAB Function55/6(L0_ddot)
  [Tp_2] -> MATLAB Function55/3(Tp)
  [T1] -> Subsystem15/1(T1)
  [T2] -> Subsystem15/2(T2)
  [T3] -> Subsystem15/3(T3)
  [T4] -> Subsystem15/4(T4)
  [Tw_1] -> Subsystem15/5(Tw1)
  Add7/1 -> [Tw_1]
  [Twy1] -> Add7/2
  [Tw1] -> Add7/1
  [Twy2] -> Add8/2
  [Tw2] -> Add8/1
  Add8/1 -> [Tw_2]
  [Tw_2] -> Subsystem15/6(Tw2)
  [F_N1] -> Subsystem15/7(FN)
  [L2] -> EKF2/3(L2)
  EKF2/2(dx_hat1) -> [dx_hat2]
  EKF2/1(x_hat1) -> [x_hat2]
  [acc_x] -> EKF2/1(acc_x)
  [dx_w] -> Unit Delay9/1
  [Tw_2] -> Unit Delay8/1
  [Tp_2] -> Unit Delay7/1
  Unit Delay4/1 -> EKF1/4(Tw1)
  Unit Delay5/1 -> EKF1/5(Tp1)
  Unit Delay7/1 -> EKF2/5(Tp2)
  Unit Delay8/1 -> EKF2/4(Tw2)
  Unit Delay2/1 -> [F_N1]
  Unit Delay3/1 -> [F_N2]
  Unit Delay6/1 -> EKF1/2(dx_w)
  Unit Delay9/1 -> EKF2/2(dx_w)
  Constant36/1 -> Mux48/1, Mux41/6, Mux47/1, Mux41/5, Mux44/1, Mux41/2, Mux43/1, Mux41/1
  [theta1] -> Mux43/2
  [dtheta1] -> Mux44/2
  [x_hat1] -> Mux45/2
  [dx_hat1] -> Mux46/2
  [phi] -> Mux47/2
  [dphi] -> Mux48/2
  [theta1] -> Fl18/2(theta1)
  [theta2] -> Fl17/4(theta2)
  Rate Limiter13/1 -> Analog Filter Design/1
  Constant37/1 -> yaw环4/2(yaw_d)
  Constant21/1 -> Rate Limiter7/1
  Integrator12/1 -> Add3/1
  Integrator11/1 -> Sum6/2
